simulink library "lib_all"  (Simulink 7.6)
settings: Solver=ode45  SolverMode=SingleTasking  StartTime=0.0  StopTime=10.0  RelTol=1e-3
values from neighbouring files (symbols this model uses):
  map_lib_03 = [6.8679 7.0159 7.4571 8.1849 9.1892 10.456 11.97 13.71 15.655 17.781 20.063 22.474 ... (803 elements, 73x11)]    [scr_simdata.mat]    (also in scr_simdata_all.mat)
  map_lib_06 = [2.5784 2.6727 2.9588 3.4453 4.1444 5.068 6.226 7.6242 9.2634 11.139 13.24 15.552 ... (803 elements, 73x11)]    [scr_simdata.mat]    (also in scr_simdata_all.mat)
  map_lib_09 = [0.0025784 0.0026727 0.0029588 0.0034453 0.0041444 0.005068 0.006226 0.0076242 0.0092634 0.011139 0.01324 0.015552 ... (803 elements, 73x11)]    [scr_simdata.mat]    (also in scr_simdata_all.mat)
  map_lib_10 = [2.74 2.75 2.76 2.77 2.78 2.79 2.8 2.81 2.82 2.83 2.84 2.85 ... (81 elements, 1x81)]    [scr_simdata.mat]    (also in scr_simdata_all.mat)
  map_lib_11 = [1.1983 1.2027 1.207 1.2113 1.2156 1.2198 1.2241 1.2283 1.2326 1.2368 1.241 1.2451 ... (81 elements, 1x81)]    [scr_simdata.mat]    (also in scr_simdata_all.mat)
  map_lib_12 = [2.74 2.75 2.76 2.77 2.78 2.79 2.8 2.81 2.82 2.83 2.84 2.85 ... (81 elements, 1x81)]    [scr_simdata.mat]    (also in scr_simdata_all.mat)
  map_lib_13 = [1.74 1.75 1.76 1.77 1.78 1.79 1.8 1.81 1.82 1.83 1.84 1.85 ... (81 elements, 1x81)]    [scr_simdata.mat]    (also in scr_simdata_all.mat)
  map_lib_16 = [0.00048828125 1.6159 1.8962 2.0821 2.2251 2.3426 2.4433 2.5318 2.611 2.683 2.749 2.8101 ... (1419 elements, 129x11)]    [scr_simdata.mat]    (also in scr_simdata_all.mat)
  map_mr2_01 = [0 800 1600 2400 3200 4000]    [scr_simdata.mat]    (also in scr_simdata_all.mat)
  map_mr2_02 = [1.32 1.32 1.33 1.34 1.36 1.38]    [scr_simdata.mat]    (also in scr_simdata_all.mat)
  map_mr2_03 = [0 800 1600 2400 3200 4000]    [scr_simdata.mat]    (also in scr_simdata_all.mat)
  map_mr2_04 = [1.32 1.32 1.33 1.34 1.36 1.38]    [scr_simdata.mat]    (also in scr_simdata_all.mat)
  map_mr2_05 = [0 800 1600 2400 3200 4000]    [scr_simdata.mat]    (also in scr_simdata_all.mat)
  map_mr2_06 = [1.32 1.32 1.33 1.34 1.36 1.38]    [scr_simdata.mat]    (also in scr_simdata_all.mat)
  Parameter1 = 6    [scr_simdata.mat]    (also in scr_simdata_all.mat)
  Parameter101 = 30    [scr_simdata.mat]    (also in scr_simdata_all.mat)
  Parameter102 = 12    [scr_simdata.mat]    (also in scr_simdata_all.mat)
  Parameter103 = -240    [scr_simdata.mat]    (also in scr_simdata_all.mat)
  Parameter108 = 48    [scr_simdata.mat]    (also in scr_simdata_all.mat)
  Parameter120 = 16    [scr_simdata.mat]    (also in scr_simdata_all.mat)
  Parameter121 = 87    [scr_simdata.mat]    (also in scr_simdata_all.mat)
  Parameter123 = 78    [scr_simdata.mat]    (also in scr_simdata_all.mat)
  Parameter124 = 60    [scr_simdata.mat]    (also in scr_simdata_all.mat)
  Parameter125 = -480    [scr_simdata.mat]    (also in scr_simdata_all.mat)
  Parameter130 = 96    [scr_simdata.mat]    (also in scr_simdata_all.mat)
  Parameter131 = 3    [scr_simdata.mat]    (also in scr_simdata_all.mat)
  Parameter144 = 3    [scr_simdata.mat]    (also in scr_simdata_all.mat)
  Parameter18 = 128    [scr_simdata.mat]    (also in scr_simdata_all.mat)
  Parameter19 = 16    [scr_simdata.mat]    (also in scr_simdata_all.mat)
  Parameter2 = 12    [scr_simdata.mat]    (also in scr_simdata_all.mat)
  Parameter20 = 7    [scr_simdata.mat]    (also in scr_simdata_all.mat)
  Parameter21 = 4    [scr_simdata.mat]    (also in scr_simdata_all.mat)
  Parameter3 = 18    [scr_simdata.mat]    (also in scr_simdata_all.mat)
  Parameter32 = 1    [scr_simdata.mat]    (also in scr_simdata_all.mat)
  Parameter34 = 24    [scr_simdata.mat]    (also in scr_simdata_all.mat)
  Parameter4 = 138    [scr_simdata.mat]    (also in scr_simdata_all.mat)
  Parameter42 = 48    [scr_simdata.mat]    (also in scr_simdata_all.mat)
  Parameter5 = 135    [scr_simdata.mat]    (also in scr_simdata_all.mat)
  Parameter55 = 0    [scr_simdata.mat]    (also in scr_simdata_all.mat)
  Parameter56 = 0    [scr_simdata.mat]    (also in scr_simdata_all.mat)
  Parameter57 = 1    [scr_simdata.mat]    (also in scr_simdata_all.mat)
  Parameter58 = 1    [scr_simdata.mat]    (also in scr_simdata_all.mat)
  Parameter59 = 2    [scr_simdata.mat]    (also in scr_simdata_all.mat)
  Parameter6 = 132    [scr_simdata.mat]    (also in scr_simdata_all.mat)
  Parameter60 = 2    [scr_simdata.mat]    (also in scr_simdata_all.mat)
  Parameter63 = 144    [scr_simdata.mat]    (also in scr_simdata_all.mat)
  Parameter7 = 126    [scr_simdata.mat]    (also in scr_simdata_all.mat)
  Parameter76 = 64    [scr_simdata.mat]    (also in scr_simdata_all.mat)
  Parameter77 = 135    [scr_simdata.mat]    (also in scr_simdata_all.mat)
  Parameter79 = 126    [scr_simdata.mat]    (also in scr_simdata_all.mat)
  Parameter80 = 108    [scr_simdata.mat]    (also in scr_simdata_all.mat)
  Parameter81 = 0    [scr_simdata.mat]    (also in scr_simdata_all.mat)
  Parameter86 = 0    [scr_simdata.mat]    (also in scr_simdata_all.mat)
  Parameter98 = 112    [scr_simdata.mat]    (also in scr_simdata_all.mat)
  Parameter99 = 39    [scr_simdata.mat]    (also in scr_simdata_all.mat)
undefined symbols (not defined in the file or in its folder): cylvolkp_hih_map2h, cylvolkp_map2h, pk1k_map2h
libraries: simulink x2
(part 5 of 36)

== system lib_all/SubSystem727/SubSystem737 ==
  Block1699 : Inport
  Block1700 : Inport  Port=2
  Block1701 : Inport  Port=3
  Block1702 : EnablePort
  Block1703 : DataStoreWrite  DataStoreName=DSM22
  Block1704 : DataStoreWrite  DataStoreName=DSM32
  Block1705 : DataStoreWrite  DataStoreName=DSM27
  wires:
  Block1699/1 -> Block1703/1
  Block1700/1 -> Block1704/1
  Block1701/1 -> Block1705/1

== system lib_all/SubSystem727/SubSystem738 ==
  Block1706 : Inport
  Block1707 : Inport  Port=2
  Block1708 : Inport  Port=3
  Block1709 : EnablePort
  Block1710 : DataStoreWrite  DataStoreName=DSM23
  Block1711 : DataStoreWrite  DataStoreName=DSM33
  Block1712 : DataStoreWrite  DataStoreName=DSM28
  wires:
  Block1706/1 -> Block1710/1
  Block1707/1 -> Block1711/1
  Block1708/1 -> Block1712/1

== system lib_all/SubSystem727/SubSystem739 ==
  Block1713 : Inport
  Block1714 : Inport  Port=2
  Block1715 : Inport  Port=3
  Block1716 : EnablePort
  Block1717 : DataStoreWrite  DataStoreName=DSM24
  Block1718 : DataStoreWrite  DataStoreName=DSM34
  Block1719 : DataStoreWrite  DataStoreName=DSM29
  wires:
  Block1715/1 -> Block1719/1
  Block1714/1 -> Block1718/1
  Block1713/1 -> Block1717/1

== system lib_all/SubSystem727/SubSystem740 ==
  note: Text113
  note: Text112
  Block1720 : Inport
  Block1721 : Inport  Port=2
  Block1722 : Inport  Port=3
  Block1723 : Constant  Value=0  OutDataTypeStr=double  SampleTime=-1
  SubSystem742 : Subsystem
  SubSystem743 : Subsystem
  SubSystem751 : Subsystem  TreatAsAtomicUnit=on
  SubSystem752 : Subsystem
  Block1778 : Outport
  wires:
  [TagName23] -> SubSystem751/3(Block1765)
  [TagName11] -> SubSystem751/2(Block1764)
  [TagName1] -> SubSystem751/1(Block1763)
  SubSystem742/1(Out1) -> SubSystem751/Enable
  Block1723/1 -> SubSystem742/2(In2)
  [TagName4] -> SubSystem742/1(In1)
  SubSystem752/1(Block1777) -> Block1778/1, [TagName23]
  Block1722/1 -> SubSystem752/1(Block1770)
  SubSystem743/1(Block1762) -> SubSystem752/2(Block1771), [TagName11]
  Block1720/1 -> SubSystem743/2(Block1733), [TagName4]
  Block1721/1 -> SubSystem743/1(Block1732), [TagName1]

== system lib_all/SubSystem727/SubSystem754 ==
  note: Text121
  note: Text120
  note: Text119
  Block1779 : Inport
  Block1780 : Inport  Port=2
  Block1781 : Inport  Port=3
  Block1782 : Constant  OutDataTypeStr=double  SampleTime=-1
  Block1783 : Gain  Gain=1/5  SaturateOnIntegerOverflow=on  OutDataTypeStr=Inherit: Same as input  ParamDataTypeStr=Inherit: Same as input
      settings: AttributesFormatString=Attribute365
  Block1784 : Product  OutDataTypeStr=double  SaturateOnIntegerOverflow=on  RndMeth=Zero  InputSameDT=on
  Block1785 : Sum  Inputs=-+  OutDataTypeStr=double  RndMeth=Zero  SaturateOnIntegerOverflow=on  InputSameDT=on
  Block1786 : Sum  OutDataTypeStr=double  RndMeth=Zero  SaturateOnIntegerOverflow=on  InputSameDT=on
  Block1787 : Sum  OutDataTypeStr=double  RndMeth=Zero  SaturateOnIntegerOverflow=on  InputSameDT=on
  SubSystem756 : Subsystem
  SubSystem762 : Subsystem
  Block1878 : Outport
  wires:
  Block1787/1 -> SubSystem762/3(Block1816), SubSystem756/2(Block1789)
  Block1779/1 -> Block1787/1
  Block1783/1 -> Block1786/2
  Block1784/1 -> Block1783/1
  Block1782/1 -> Block1787/2
  Block1786/1 -> Block1878/1
  SubSystem756/2(Block1813) -> Block1784/2
  Block1785/1 -> Block1784/1  "SigLabel48"
  SubSystem762/2(Block1877) -> Block1785/2
  SubSystem762/1(Block1876) -> Block1785/1, Block1786/1
  Block1781/1 -> SubSystem762/2(Block1815)
  SubSystem756/1(Block1812) -> SubSystem762/1(Block1814)
  Block1780/1 -> SubSystem756/1(Block1788)

== system lib_all/SubSystem795/SubSystem796 ==
  note: ***編集禁止***
  In1 : Inport
  In2 : Inport  Port=2
  RO1 : RelationalOperator  Operator=>  InputSameDT=on
  single1 : DataTypeConversion  OutDataTypeStr=single  SaturateOnIntegerOverflow=on  RndMeth=Zero
  single2 : DataTypeConversion  OutDataTypeStr=single  SaturateOnIntegerOverflow=on  RndMeth=Zero
  Out1 : Outport
  wires:
  RO1/1 -> Out1/1
  In2/1 -> single2/1
  In1/1 -> single1/1
  single1/1 -> RO1/1
  single2/1 -> RO1/2

== system lib_all/SubSystem795/SubSystem797 ==
  note: ***編集禁止***
  In1 : Inport
  In2 : Inport  Port=2
  RO1 : RelationalOperator  Operator=>  InputSameDT=on
  single1 : DataTypeConversion  OutDataTypeStr=single  SaturateOnIntegerOverflow=on  RndMeth=Zero
  single2 : DataTypeConversion  OutDataTypeStr=single  SaturateOnIntegerOverflow=on  RndMeth=Zero
  Out1 : Outport
  wires:
  single2/1 -> RO1/2
  single1/1 -> RO1/1
  In1/1 -> single1/1
  In2/1 -> single2/1
  RO1/1 -> Out1/1

== system lib_all/SubSystem795/SubSystem798 ==
  note: ***編集禁止***
  In1 : Inport
  In2 : Inport  Port=2
  LO1 : Logic
  RO1 : RelationalOperator  Operator=<  InputSameDT=on
  RO2 : RelationalOperator  Operator=~=  InputSameDT=on
  single1 : DataTypeConversion  OutDataTypeStr=single  SaturateOnIntegerOverflow=on  RndMeth=Zero
  single2 : DataTypeConversion  OutDataTypeStr=single  SaturateOnIntegerOverflow=on  RndMeth=Zero
  Out1 : Outport
  wires:
  single2/1 -> RO1/2, RO2/2
  single1/1 -> RO1/1, RO2/1
  In1/1 -> single1/1
  In2/1 -> single2/1
  RO1/1 -> LO1/1
  RO2/1 -> LO1/2
  LO1/1 -> Out1/1

== system lib_all/SubSystem795/SubSystem799 ==
  note: ***編集禁止***
  In1 : Inport
  In2 : Inport  Port=2
  LO1 : Logic
  RO1 : RelationalOperator  Operator=<  InputSameDT=on
  RO2 : RelationalOperator  Operator=~=  InputSameDT=on
  single1 : DataTypeConversion  OutDataTypeStr=single  SaturateOnIntegerOverflow=on  RndMeth=Zero
  single2 : DataTypeConversion  OutDataTypeStr=single  SaturateOnIntegerOverflow=on  RndMeth=Zero
  Out1 : Outport
  wires:
  single2/1 -> RO1/2, RO2/2
  single1/1 -> RO1/1, RO2/1
  In1/1 -> single1/1
  In2/1 -> single2/1
  RO1/1 -> LO1/1
  RO2/1 -> LO1/2
  LO1/1 -> Out1/1

== system lib_all/SubSystem800/SubSystem801 ==
  note: ***編集禁止***
  element : Inport
  Inside_DSR : DataStoreRead  DataStoreName=DSM182
  Selector : Selector  IndexOptions=Index vector (port)  Indices=[1 3]  OutputSizes=1
  Output : Outport
  wires:
  Inside_DSR/1 -> Selector/1
  element/1 -> Selector/2
  Selector/1 -> Output/1

== system lib_all/SubSystem800/SubSystem802 ==
  note: ***編集禁止***
  In1 : Inport
  In2 : Inport  Port=2
  LO1 : Logic
  RO1 : RelationalOperator  Operator=<  InputSameDT=on
  RO2 : RelationalOperator  Operator=~=  InputSameDT=on
  single1 : DataTypeConversion  OutDataTypeStr=single  SaturateOnIntegerOverflow=on  RndMeth=Zero
  single2 : DataTypeConversion  OutDataTypeStr=single  SaturateOnIntegerOverflow=on  RndMeth=Zero
  Out1 : Outport
  wires:
  In2/1 -> single2/1
  In1/1 -> single1/1
  single1/1 -> RO1/1, RO2/1
  single2/1 -> RO1/2, RO2/2
  RO1/1 -> LO1/1
  RO2/1 -> LO1/2
  LO1/1 -> Out1/1

== system lib_all/SubSystem800/SubSystem803 ==
  note: Text137
  note: Text136
  note: Text135
  note: Text134
  note: Text133
  Block1916 : Inport
  Block1917 : Inport  Port=2
  Block1918 : Constant  Value=Parameter81  OutDataTypeStr=double  SampleTime=-1
  Block1919 : Constant  Value=Parameter103  OutDataTypeStr=double  SampleTime=-1
  Block1920 : Constant  Value=Parameter125  OutDataTypeStr=double  SampleTime=-1
  Block1921 : Constant  Value=143  OutDataTypeStr=double  SampleTime=-1
  Block1922 : Constant  Value=-3  OutDataTypeStr=double  SampleTime=-1
  Block1923 : Constant  Value=359  OutDataTypeStr=double  SampleTime=-1
  Block1924 : Constant  Value=359  OutDataTypeStr=double  SampleTime=-1
  Block1925 : DataStoreRead  DataStoreName=DSM50
  Block1926 : Gain  Gain=5  SaturateOnIntegerOverflow=on  OutDataTypeStr=Inherit: Same as input  ParamDataTypeStr=Inherit: Same as input
      settings: AttributesFormatString=Attribute395
  Block1927 : Ground
  Block1928 : MultiPortSwitch  Inputs=4  zeroidx=off  RndMeth=Zero  SaturateOnIntegerOverflow=on  InputSameDT=on
  SubSystem804 : Subsystem  TreatAsAtomicUnit=on
      settings: AttributesFormatString=Attribute396  RTWFcnNameOpts=Use subsystem name
  SubSystem809 : Subsystem  TreatAsAtomicUnit=on
      settings: AttributesFormatString=Attribute403  RTWFcnNameOpts=Use subsystem name
  SubSystem814 : Subsystem  TreatAsAtomicUnit=on
      settings: AttributesFormatString=Attribute410  RTWFcnNameOpts=Use subsystem name
  Block2001 : Outport
  Block2002 : Outport  Port=2
  wires:
  Block1916/1 -> Block1928/1
  Block1918/1 -> Block1928/2
  Block1919/1 -> Block1928/3
  Block1920/1 -> Block1928/4
  Block1927/1 -> Block1928/5
  Block1928/1 -> SubSystem814/3(Block1979)  "SigLabel55"
  Block1921/1 -> SubSystem804/1(Block1929)
  Block1917/1 -> SubSystem804/2(Block1930), Block1926/1
  Block1922/1 -> SubSystem804/3(Block1931)
  Block1926/1 -> SubSystem809/2(Block1954)
  Block1923/1 -> SubSystem809/1(Block1953)
  Block1925/1 -> SubSystem809/3(Block1955)
  Block1924/1 -> SubSystem814/1(Block1977)
  SubSystem809/1(Block1976) -> SubSystem814/2(Block1978)
  SubSystem804/1(Block1952) -> Block2001/1
  SubSystem814/1(Block2000) -> Block2002/1

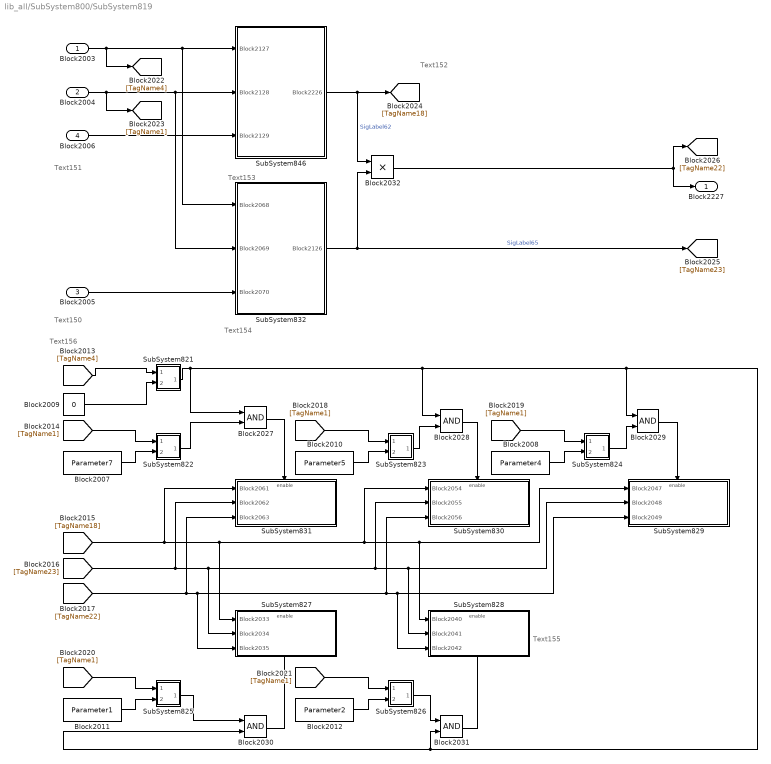
== system lib_all/SubSystem800/SubSystem819 ==
  note: Text156
  note: Text155
  note: Text154
  note: Text153
  note: Text152
  note: Text151
  note: Text150
  Block2003 : Inport
  Block2004 : Inport  Port=2
  Block2005 : Inport  Port=3
  Block2006 : Inport  Port=4
  Block2007 : Constant  Value=Parameter7  OutDataTypeStr=double  SampleTime=-1
  Block2008 : Constant  Value=Parameter4  OutDataTypeStr=double  SampleTime=-1
  Block2009 : Constant  Value=0  OutDataTypeStr=double  SampleTime=-1
  Block2010 : Constant  Value=Parameter5  OutDataTypeStr=double  SampleTime=-1
  Block2011 : Constant  Value=Parameter1  OutDataTypeStr=double  SampleTime=-1
  Block2012 : Constant  Value=Parameter2  OutDataTypeStr=double  SampleTime=-1
  Block{2027..2031} (5 alike) : Logic
  Block2032 : Product  OutDataTypeStr=double  SaturateOnIntegerOverflow=on  RndMeth=Zero  InputSameDT=on
  SubSystem821 : Subsystem
  SubSystem822 : Subsystem
  SubSystem823 : Subsystem
  SubSystem824 : Subsystem
  SubSystem825 : Subsystem
  SubSystem826 : Subsystem
  SubSystem827 : Subsystem
      settings: PropExecContextOutsideSubsystem=on
  SubSystem828 : Subsystem
      settings: PropExecContextOutsideSubsystem=on
  SubSystem829 : Subsystem
      settings: PropExecContextOutsideSubsystem=on
  SubSystem830 : Subsystem
      settings: PropExecContextOutsideSubsystem=on
  SubSystem831 : Subsystem
      settings: PropExecContextOutsideSubsystem=on
  SubSystem832 : Subsystem  Priority=2  TreatAsAtomicUnit=on
      settings: AttributesFormatString=Attribute424  RTWFcnNameOpts=Use subsystem name
  SubSystem846 : Subsystem  Priority=1  TreatAsAtomicUnit=on
      settings: AttributesFormatString=Attribute438  RTWFcnNameOpts=Use subsystem name
  Block2227 : Outport
  wires:
  SubSystem826/1(Out1) -> Block2031/1
  SubSystem825/1(Out1) -> Block2030/1
  Block2031/1 -> SubSystem828/Enable
  Block2030/1 -> SubSystem827/Enable
  Block2012/1 -> SubSystem826/2(In2)
  [TagName1] -> SubSystem826/1(In1)
  Block2011/1 -> SubSystem825/2(In2)
  [TagName1] -> SubSystem825/1(In1)
  [TagName22] -> SubSystem831/3(Block2063), SubSystem827/3(Block2035), SubSystem829/3(Block2049), SubSystem828/3(Block2042), SubSystem830/3(Block2056)
  [TagName23] -> SubSystem831/2(Block2062), SubSystem827/2(Block2034), SubSystem829/2(Block2048), SubSystem828/2(Block2041), SubSystem830/2(Block2055)
  [TagName18] -> SubSystem831/1(Block2061), SubSystem827/1(Block2033), SubSystem829/1(Block2047), SubSystem828/1(Block2040), SubSystem830/1(Block2054)
  Block2029/1 -> SubSystem829/Enable
  Block2028/1 -> SubSystem830/Enable
  SubSystem824/1(Out1) -> Block2029/2
  Block2008/1 -> SubSystem824/2(In2)
  [TagName1] -> SubSystem824/1(In1)
  Block2027/1 -> SubSystem831/Enable
  SubSystem823/1(Out1) -> Block2028/2
  Block2010/1 -> SubSystem823/2(In2)
  [TagName1] -> SubSystem823/1(In1)
  SubSystem822/1(Out1) -> Block2027/2
  SubSystem821/1(Out1) -> Block2027/1, Block2028/1, Block2029/1, Block2031/2, Block2030/2
  Block2007/1 -> SubSystem822/2(In2)
  [TagName1] -> SubSystem822/1(In1)
  Block2009/1 -> SubSystem821/2(In2)
  [TagName4] -> SubSystem821/1(In1)
  Block2032/1 -> Block2227/1, [TagName22]
  SubSystem832/1(Block2126) -> Block2032/2, [TagName23]  "SigLabel65"
  SubSystem846/1(Block2226) -> Block2032/1, [TagName18]  "SigLabel62"
  Block2004/1 -> SubSystem832/2(Block2069), SubSystem846/2(Block2128), [TagName1]
  Block2003/1 -> SubSystem832/1(Block2068), SubSystem846/1(Block2127), [TagName4]
  Block2006/1 -> SubSystem846/3(Block2129)
  Block2005/1 -> SubSystem832/3(Block2070)

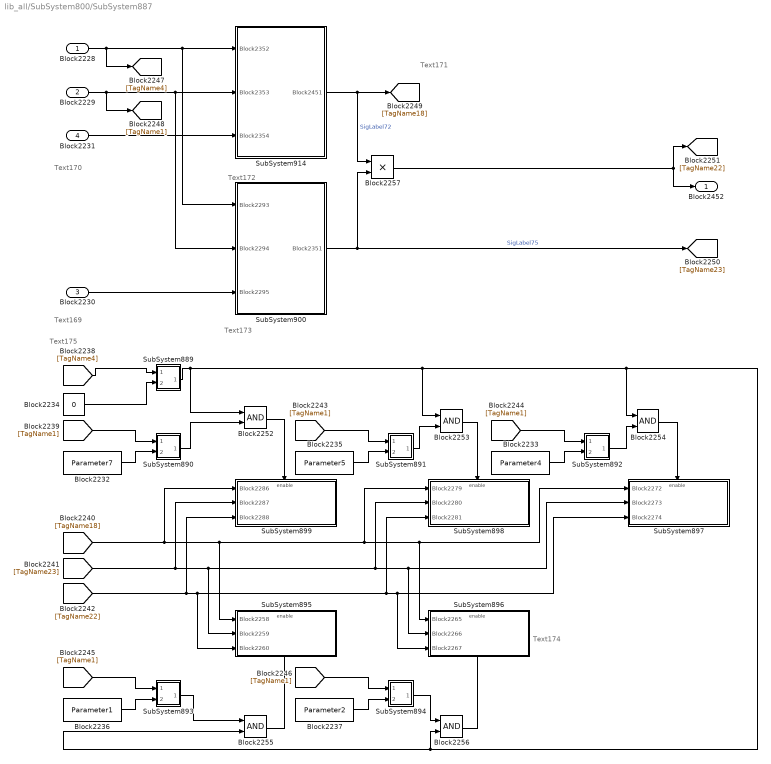
== system lib_all/SubSystem800/SubSystem887 ==
  note: Text175
  note: Text174
  note: Text173
  note: Text172
  note: Text171
  note: Text170
  note: Text169
  Block2228 : Inport
  Block2229 : Inport  Port=2
  Block2230 : Inport  Port=3
  Block2231 : Inport  Port=4
  Block2232 : Constant  Value=Parameter7  OutDataTypeStr=double  SampleTime=-1
  Block2233 : Constant  Value=Parameter4  OutDataTypeStr=double  SampleTime=-1
  Block2234 : Constant  Value=0  OutDataTypeStr=double  SampleTime=-1
  Block2235 : Constant  Value=Parameter5  OutDataTypeStr=double  SampleTime=-1
  Block2236 : Constant  Value=Parameter1  OutDataTypeStr=double  SampleTime=-1
  Block2237 : Constant  Value=Parameter2  OutDataTypeStr=double  SampleTime=-1
  Block{2252..2256} (5 alike) : Logic
  Block2257 : Product  OutDataTypeStr=double  SaturateOnIntegerOverflow=on  RndMeth=Zero  InputSameDT=on
  SubSystem889 : Subsystem
  SubSystem890 : Subsystem
  SubSystem891 : Subsystem
  SubSystem892 : Subsystem
  SubSystem893 : Subsystem
  SubSystem894 : Subsystem
  SubSystem895 : Subsystem
      settings: PropExecContextOutsideSubsystem=on
  SubSystem896 : Subsystem
      settings: PropExecContextOutsideSubsystem=on
  SubSystem897 : Subsystem
      settings: PropExecContextOutsideSubsystem=on
  SubSystem898 : Subsystem
      settings: PropExecContextOutsideSubsystem=on
  SubSystem899 : Subsystem
      settings: PropExecContextOutsideSubsystem=on
  SubSystem900 : Subsystem  Priority=2  TreatAsAtomicUnit=on
      settings: AttributesFormatString=Attribute461  RTWFcnNameOpts=Use subsystem name
  SubSystem914 : Subsystem  Priority=1  TreatAsAtomicUnit=on
      settings: AttributesFormatString=Attribute475  RTWFcnNameOpts=Use subsystem name
  Block2452 : Outport
  wires:
  SubSystem894/1(Out1) -> Block2256/1
  SubSystem893/1(Out1) -> Block2255/1
  Block2256/1 -> SubSystem896/Enable
  Block2255/1 -> SubSystem895/Enable
  Block2237/1 -> SubSystem894/2(In2)
  [TagName1] -> SubSystem894/1(In1)
  Block2236/1 -> SubSystem893/2(In2)
  [TagName1] -> SubSystem893/1(In1)
  [TagName22] -> SubSystem899/3(Block2288), SubSystem895/3(Block2260), SubSystem897/3(Block2274), SubSystem896/3(Block2267), SubSystem898/3(Block2281)
  [TagName23] -> SubSystem899/2(Block2287), SubSystem895/2(Block2259), SubSystem897/2(Block2273), SubSystem896/2(Block2266), SubSystem898/2(Block2280)
  [TagName18] -> SubSystem899/1(Block2286), SubSystem895/1(Block2258), SubSystem897/1(Block2272), SubSystem896/1(Block2265), SubSystem898/1(Block2279)
  Block2254/1 -> SubSystem897/Enable
  Block2253/1 -> SubSystem898/Enable
  SubSystem892/1(Out1) -> Block2254/2
  Block2233/1 -> SubSystem892/2(In2)
  [TagName1] -> SubSystem892/1(In1)
  Block2252/1 -> SubSystem899/Enable
  SubSystem891/1(Out1) -> Block2253/2
  Block2235/1 -> SubSystem891/2(In2)
  [TagName1] -> SubSystem891/1(In1)
  SubSystem890/1(Out1) -> Block2252/2
  SubSystem889/1(Out1) -> Block2252/1, Block2253/1, Block2254/1, Block2256/2, Block2255/2
  Block2232/1 -> SubSystem890/2(In2)
  [TagName1] -> SubSystem890/1(In1)
  Block2234/1 -> SubSystem889/2(In2)
  [TagName4] -> SubSystem889/1(In1)
  Block2257/1 -> Block2452/1, [TagName22]
  SubSystem900/1(Block2351) -> Block2257/2, [TagName23]  "SigLabel75"
  SubSystem914/1(Block2451) -> Block2257/1, [TagName18]  "SigLabel72"
  Block2229/1 -> SubSystem900/2(Block2294), SubSystem914/2(Block2353), [TagName1]
  Block2228/1 -> SubSystem900/1(Block2293), SubSystem914/1(Block2352), [TagName4]
  Block2231/1 -> SubSystem914/3(Block2354)
  Block2230/1 -> SubSystem900/3(Block2295)

== system lib_all/SubSystem800/SubSystem955 ==
  Block2453 : Inport
  Block2454 : Inport  Port=2
  Block2455 : Inport  Port=3
  Block2456 : Inport  Port=4
  Block2457 : EnablePort
  SubSystem956 : Subsystem  TreatAsAtomicUnit=on  mask(***ATC.DSWVEC***){bd_version=1.01.A, r0=DSM182, r1=-1, r2=, r3=, r4=, r5=, r6=, r7=, r8=, r9=, c0=, c1=, c2=, c3=, c4=, c5=, c6=, c7=, c8=, c9=}
      mask prompts: bd_version "Version"
      mask help: これはAutoCからのブロックです．編集禁止．
      settings: OpenFcn=warndlg('変更禁止!');
      mask Help: web(ui_blockhelpmng(gcb));
      mask Display: disp('ATC.DSRVEC')
  SubSystem957 : Subsystem  TreatAsAtomicUnit=on  mask(***ATC.DSWVEC***){bd_version=1.01.A, r0=DSM184, r1=-1, r2=, r3=, r4=, r5=, r6=, r7=, r8=, r9=, c0=, c1=, c2=, c3=, c4=, c5=, c6=, c7=, c8=, c9=}
      mask prompts: bd_version "Version"
      mask help: これはAutoCからのブロックです．編集禁止．
      settings: OpenFcn=warndlg('変更禁止!');
      mask Help: web(ui_blockhelpmng(gcb));
      mask Display: disp('ATC.DSRVEC')
  SubSystem958 : Subsystem  TreatAsAtomicUnit=on  mask(***ATC.DSWVEC***){bd_version=1.01.A, r0=DSM183, r1=-1, r2=, r3=, r4=, r5=, r6=, r7=, r8=, r9=, c0=, c1=, c2=, c3=, c4=, c5=, c6=, c7=, c8=, c9=}
      mask prompts: bd_version "Version"
      mask help: これはAutoCからのブロックです．編集禁止．
      settings: OpenFcn=warndlg('変更禁止!');
      mask Help: web(ui_blockhelpmng(gcb));
      mask Display: disp('ATC.DSRVEC')
  wires:
  Block2456/1 -> SubSystem958/1(input)
  Block2455/1 -> SubSystem957/1(input)
  Block2454/1 -> SubSystem956/1(input)
  Block2453/1 -> SubSystem957/2(element), SubSystem958/2(element), SubSystem956/2(element)

== system lib_all/SubSystem959/SubSystem961 ==
  note: ***編集禁止***
  N : Inport
  For_loop : Stateflow chart  TreatAsAtomicUnit=on
  action : Outport
  i : Outport  Port=2
  wires:
  For_loop/2(function_call) -> action/1
  N/1 -> For_loop/1(N_Loop)
  For_loop/1(idx) -> i/1

== system lib_all/SubSystem959/SubSystem963 ==
  note: Text186
  Block2469 : Inport
  Block2470 : Inport  Port=2
  Block2471 : Inport  Port=3
  Block2472 : TriggerPort  TriggerType=function-call
  Block2473 : Constant  Value=2  OutDataTypeStr=double  SampleTime=-1
  Block2474 : Constant  OutDataTypeStr=double  SampleTime=-1
  Block2475 : Constant  Value=144  OutDataTypeStr=double  SampleTime=-1
  Block2476 : Constant  Value=0  OutDataTypeStr=double  SampleTime=-1
  Block2477 : Constant  Value=0  OutDataTypeStr=double  SampleTime=-1
  Block2478 : Constant  OutDataTypeStr=double  SampleTime=-1
  Block2479 : Constant  OutDataTypeStr=double  SampleTime=-1
  Block2480 : Sum  Inputs=+-  OutDataTypeStr=double  RndMeth=Zero  SaturateOnIntegerOverflow=on  InputSameDT=on
  Block{2481..2485} (5 alike) : Sum  OutDataTypeStr=double  RndMeth=Zero  SaturateOnIntegerOverflow=on  InputSameDT=on
  Block2486 : Switch  Threshold=0.5  RndMeth=Zero  SaturateOnIntegerOverflow=on  InputSameDT=on
  Block2487 : Switch  Threshold=0.5  RndMeth=Zero  SaturateOnIntegerOverflow=on  InputSameDT=on
  Block2488 : UnitDelay  SampleTime=-1
  Block2489 : UnitDelay  SampleTime=-1
  SubSystem1018 : Subsystem
      settings: RTWSystemCode=Inline
  SubSystem1021 : Subsystem  TreatAsAtomicUnit=on  mask(***ATC.DSRVEC***){bd_version=1.01.A, r0=DSM49, r1=-1, r2=, r3=, r4=, r5=, r6=, r7=, r8=, r9=, c0=, c1=, c2=, c3=, c4=, c5=, c6=, c7=, c8=, c9=}
      mask prompts: bd_version "Version"
      mask help: これはAutoCからのブロックです．編集禁止．
      settings: OpenFcn=warndlg('変更禁止!');
      mask Help: web(ui_blockhelpmng(gcb));
      mask Display: disp('ATC.DSRVEC')
  SubSystem1022 : Subsystem  TreatAsAtomicUnit=on  mask(***ATC.DSRVEC***){bd_version=1.01.A, r0=DSM36, r1=-1, r2=, r3=, r4=, r5=, r6=, r7=, r8=, r9=, c0=, c1=, c2=, c3=, c4=, c5=, c6=, c7=, c8=, c9=}
      mask prompts: bd_version "Version"
      mask help: これはAutoCからのブロックです．編集禁止．
      settings: OpenFcn=warndlg('変更禁止!');
      mask Help: web(ui_blockhelpmng(gcb));
      mask Display: disp('ATC.DSRVEC')
  SubSystem964 : Subsystem
  SubSystem965 : Subsystem  TreatAsAtomicUnit=on
      settings: AttributesFormatString=Attribute504  RTWFcnNameOpts=Use subsystem name
  Block2620 : Outport
  Block2621 : Outport  Port=2
  wires:
  Block2486/1 -> Block2620/1, Block2489/1
  Block2473/1 -> Block2480/2
  Block2480/1 -> Block2481/1
  Block2481/1 -> SubSystem1018/2(Block2613)
  Block2475/1 -> SubSystem1018/1(Block2612)
  Block2476/1 -> SubSystem1018/3(Block2614)
  Block2471/1 -> SubSystem965/1(Block2490), Block2485/1
  Block2477/1 -> SubSystem965/4(Block2493)
  SubSystem1022/1(Output) -> SubSystem965/3(Block2492)
  Block2469/1 -> Block2480/1, SubSystem964/2(In2)
  Block2470/1 -> Block2481/2
  SubSystem965/1(Block2611) -> Block2487/1, Block2482/1
  Block2488/1 -> Block2482/2
  SubSystem1021/1(Output) -> Block2486/1, Block2483/1
  Block2489/1 -> Block2483/2
  SubSystem1018/1(Block2619) -> SubSystem965/2(Block2491), Block2484/1
  Block2485/1 -> SubSystem1022/1(element)
  Block2484/1 -> SubSystem1021/1(element)
  Block2474/1 -> SubSystem964/1(In1)
  SubSystem964/1(Out1) -> Block2486/2, Block2487/2
  Block2483/1 -> Block2486/3
  Block2482/1 -> Block2487/3
  Block2487/1 -> Block2621/1, Block2488/1
  Block2478/1 -> Block2484/2
  Block2479/1 -> Block2485/2

== system lib_all/SubSystem1023/SubSystem1025/SubSystem1027 ==
  note: ***編集禁止***
  In1 : Inport
  In2 : Inport  Port=2
  RO1 : RelationalOperator  Operator===  InputSameDT=on
  single1 : DataTypeConversion  OutDataTypeStr=single  SaturateOnIntegerOverflow=on  RndMeth=Zero
  single2 : DataTypeConversion  OutDataTypeStr=single  SaturateOnIntegerOverflow=on  RndMeth=Zero
  Out1 : Outport
  wires:
  single2/1 -> RO1/2
  single1/1 -> RO1/1
  In1/1 -> single1/1
  In2/1 -> single2/1
  RO1/1 -> Out1/1

== system lib_all/SubSystem1023/SubSystem1025/SubSystem1028 ==
  note: ***編集禁止***
  In1 : Inport
  In2 : Inport  Port=2
  RO1 : RelationalOperator  Operator===  InputSameDT=on
  single1 : DataTypeConversion  OutDataTypeStr=single  SaturateOnIntegerOverflow=on  RndMeth=Zero
  single2 : DataTypeConversion  OutDataTypeStr=single  SaturateOnIntegerOverflow=on  RndMeth=Zero
  Out1 : Outport
  wires:
  single2/1 -> RO1/2
  single1/1 -> RO1/1
  In1/1 -> single1/1
  In2/1 -> single2/1
  RO1/1 -> Out1/1

== system lib_all/SubSystem1023/SubSystem1025/SubSystem1029 ==
  note: ***編集禁止***
  In1 : Inport
  In2 : Inport  Port=2
  RO1 : RelationalOperator  Operator===  InputSameDT=on
  single1 : DataTypeConversion  OutDataTypeStr=single  SaturateOnIntegerOverflow=on  RndMeth=Zero
  single2 : DataTypeConversion  OutDataTypeStr=single  SaturateOnIntegerOverflow=on  RndMeth=Zero
  Out1 : Outport
  wires:
  single2/1 -> RO1/2
  single1/1 -> RO1/1
  In1/1 -> single1/1
  In2/1 -> single2/1
  RO1/1 -> Out1/1

== system lib_all/SubSystem1023/SubSystem1025/SubSystem1030 ==
  note: ***編集禁止***
  In1 : Inport
  In2 : Inport  Port=2
  RO1 : RelationalOperator  Operator===  InputSameDT=on
  single1 : DataTypeConversion  OutDataTypeStr=single  SaturateOnIntegerOverflow=on  RndMeth=Zero
  single2 : DataTypeConversion  OutDataTypeStr=single  SaturateOnIntegerOverflow=on  RndMeth=Zero
  Out1 : Outport
  wires:
  single2/1 -> RO1/2
  single1/1 -> RO1/1
  In1/1 -> single1/1
  In2/1 -> single2/1
  RO1/1 -> Out1/1

== system lib_all/SubSystem1023/SubSystem1025/SubSystem1031 ==
  note: ***編集禁止***
  In1 : Inport
  In2 : Inport  Port=2
  RO1 : RelationalOperator  Operator===  InputSameDT=on
  single1 : DataTypeConversion  OutDataTypeStr=single  SaturateOnIntegerOverflow=on  RndMeth=Zero
  single2 : DataTypeConversion  OutDataTypeStr=single  SaturateOnIntegerOverflow=on  RndMeth=Zero
  Out1 : Outport
  wires:
  single2/1 -> RO1/2
  single1/1 -> RO1/1
  In1/1 -> single1/1
  In2/1 -> single2/1
  RO1/1 -> Out1/1

== system lib_all/SubSystem1023/SubSystem1025/SubSystem1032 ==
  note: ***編集禁止***
  In1 : Inport
  In2 : Inport  Port=2
  RO1 : RelationalOperator  Operator===  InputSameDT=on
  single1 : DataTypeConversion  OutDataTypeStr=single  SaturateOnIntegerOverflow=on  RndMeth=Zero
  single2 : DataTypeConversion  OutDataTypeStr=single  SaturateOnIntegerOverflow=on  RndMeth=Zero
  Out1 : Outport
  wires:
  single2/1 -> RO1/2
  single1/1 -> RO1/1
  In1/1 -> single1/1
  In2/1 -> single2/1
  RO1/1 -> Out1/1

== system lib_all/SubSystem1023/SubSystem1025/SubSystem1033 ==
  Block2660 : Inport
  Block2661 : Inport  Port=2
  Block2662 : Inport  Port=3
  Block2663 : EnablePort
  Block2664 : DataStoreWrite  DataStoreName=DSM20
  Block2665 : DataStoreWrite  DataStoreName=DSM30
  Block2666 : DataStoreWrite  DataStoreName=DSM25
  wires:
  Block2660/1 -> Block2664/1
  Block2661/1 -> Block2665/1
  Block2662/1 -> Block2666/1

== system lib_all/SubSystem1023/SubSystem1025/SubSystem1034 ==
  Block2667 : Inport
  Block2668 : Inport  Port=2
  Block2669 : Inport  Port=3
  Block2670 : EnablePort
  Block2671 : DataStoreWrite  DataStoreName=DSM21
  Block2672 : DataStoreWrite  DataStoreName=DSM31
  Block2673 : DataStoreWrite  DataStoreName=DSM26
  wires:
  Block2667/1 -> Block2671/1
  Block2668/1 -> Block2672/1
  Block2669/1 -> Block2673/1

== system lib_all/SubSystem1023/SubSystem1025/SubSystem1035 ==
  Block2674 : Inport
  Block2675 : Inport  Port=2
  Block2676 : Inport  Port=3
  Block2677 : EnablePort
  Block2678 : DataStoreWrite  DataStoreName=DSM22
  Block2679 : DataStoreWrite  DataStoreName=DSM32
  Block2680 : DataStoreWrite  DataStoreName=DSM27
  wires:
  Block2674/1 -> Block2678/1
  Block2675/1 -> Block2679/1
  Block2676/1 -> Block2680/1

== system lib_all/SubSystem1023/SubSystem1025/SubSystem1036 ==
  Block2681 : Inport
  Block2682 : Inport  Port=2
  Block2683 : Inport  Port=3
  Block2684 : EnablePort
  Block2685 : DataStoreWrite  DataStoreName=DSM23
  Block2686 : DataStoreWrite  DataStoreName=DSM33
  Block2687 : DataStoreWrite  DataStoreName=DSM28
  wires:
  Block2681/1 -> Block2685/1
  Block2682/1 -> Block2686/1
  Block2683/1 -> Block2687/1

== system lib_all/SubSystem1023/SubSystem1025/SubSystem1037 ==
  Block2688 : Inport
  Block2689 : Inport  Port=2
  Block2690 : Inport  Port=3
  Block2691 : EnablePort
  Block2692 : DataStoreWrite  DataStoreName=DSM24
  Block2693 : DataStoreWrite  DataStoreName=DSM34
  Block2694 : DataStoreWrite  DataStoreName=DSM29
  wires:
  Block2690/1 -> Block2694/1
  Block2689/1 -> Block2693/1
  Block2688/1 -> Block2692/1

== system lib_all/SubSystem1023/SubSystem1025/SubSystem1038 ==
  note: Text192
  note: Text191
  Block2695 : Inport
  Block2696 : Inport  Port=2
  Block2697 : Inport  Port=3
  Block2698 : Constant  Value=0  OutDataTypeStr=double  SampleTime=-1
  SubSystem1040 : Subsystem
  SubSystem1041 : Subsystem
  SubSystem1049 : Subsystem  TreatAsAtomicUnit=on
  SubSystem1050 : Subsystem
  Block2753 : Outport
  wires:
  Block2696/1 -> [TagName1], SubSystem1041/1(Block2707)
  Block2695/1 -> [TagName4], SubSystem1041/2(Block2708)
  SubSystem1041/1(Block2737) -> [TagName11], SubSystem1050/2(Block2746)
  Block2697/1 -> SubSystem1050/1(Block2745)
  SubSystem1050/1(Block2752) -> [TagName23], Block2753/1
  [TagName4] -> SubSystem1040/1(In1)
  Block2698/1 -> SubSystem1040/2(In2)
  SubSystem1040/1(Out1) -> SubSystem1049/Enable
  [TagName1] -> SubSystem1049/1(Block2738)
  [TagName11] -> SubSystem1049/2(Block2739)
  [TagName23] -> SubSystem1049/3(Block2740)

== system lib_all/SubSystem1023/SubSystem1025/SubSystem1052 ==
  note: Text200
  note: Text199
  note: Text198
  Block2754 : Inport
  Block2755 : Inport  Port=2
  Block2756 : Inport  Port=3
  Block2757 : Constant  OutDataTypeStr=double  SampleTime=-1
  Block2758 : Gain  Gain=1/5  SaturateOnIntegerOverflow=on  OutDataTypeStr=Inherit: Same as input  ParamDataTypeStr=Inherit: Same as input
      settings: AttributesFormatString=Attribute549
  Block2759 : Product  OutDataTypeStr=double  SaturateOnIntegerOverflow=on  RndMeth=Zero  InputSameDT=on
  Block2760 : Sum  Inputs=-+  OutDataTypeStr=double  RndMeth=Zero  SaturateOnIntegerOverflow=on  InputSameDT=on
  Block2761 : Sum  OutDataTypeStr=double  RndMeth=Zero  SaturateOnIntegerOverflow=on  InputSameDT=on
  Block2762 : Sum  OutDataTypeStr=double  RndMeth=Zero  SaturateOnIntegerOverflow=on  InputSameDT=on
  SubSystem1054 : Subsystem
  SubSystem1060 : Subsystem
  Block2853 : Outport
  wires:
  Block2755/1 -> SubSystem1054/1(Block2763)
  SubSystem1054/1(Block2787) -> SubSystem1060/1(Block2789)
  Block2756/1 -> SubSystem1060/2(Block2790)
  SubSystem1060/1(Block2851) -> Block2761/1, Block2760/1
  SubSystem1060/2(Block2852) -> Block2760/2
  Block2760/1 -> Block2759/1  "SigLabel89"
  SubSystem1054/2(Block2788) -> Block2759/2
  Block2761/1 -> Block2853/1
  Block2757/1 -> Block2762/2
  Block2759/1 -> Block2758/1
  Block2758/1 -> Block2761/2
  Block2754/1 -> Block2762/1
  Block2762/1 -> SubSystem1054/2(Block2764), SubSystem1060/3(Block2791)

== system lib_all/SubSystem1023/SubSystem1093/SubSystem1095 ==
  note: ***編集禁止***
  In1 : Inport
  In2 : Inport  Port=2
  RO1 : RelationalOperator  Operator===  InputSameDT=on
  single1 : DataTypeConversion  OutDataTypeStr=single  SaturateOnIntegerOverflow=on  RndMeth=Zero
  single2 : DataTypeConversion  OutDataTypeStr=single  SaturateOnIntegerOverflow=on  RndMeth=Zero
  Out1 : Outport
  wires:
  single2/1 -> RO1/2
  single1/1 -> RO1/1
  In1/1 -> single1/1
  In2/1 -> single2/1
  RO1/1 -> Out1/1

== system lib_all/SubSystem1023/SubSystem1093/SubSystem1096 ==
  note: ***編集禁止***
  In1 : Inport
  In2 : Inport  Port=2
  RO1 : RelationalOperator  Operator===  InputSameDT=on
  single1 : DataTypeConversion  OutDataTypeStr=single  SaturateOnIntegerOverflow=on  RndMeth=Zero
  single2 : DataTypeConversion  OutDataTypeStr=single  SaturateOnIntegerOverflow=on  RndMeth=Zero
  Out1 : Outport
  wires:
  single2/1 -> RO1/2
  single1/1 -> RO1/1
  In1/1 -> single1/1
  In2/1 -> single2/1
  RO1/1 -> Out1/1

== system lib_all/SubSystem1023/SubSystem1093/SubSystem1097 ==
  note: ***編集禁止***
  In1 : Inport
  In2 : Inport  Port=2
  RO1 : RelationalOperator  Operator===  InputSameDT=on
  single1 : DataTypeConversion  OutDataTypeStr=single  SaturateOnIntegerOverflow=on  RndMeth=Zero
  single2 : DataTypeConversion  OutDataTypeStr=single  SaturateOnIntegerOverflow=on  RndMeth=Zero
  Out1 : Outport
  wires:
  single2/1 -> RO1/2
  single1/1 -> RO1/1
  In1/1 -> single1/1
  In2/1 -> single2/1
  RO1/1 -> Out1/1

== system lib_all/SubSystem1023/SubSystem1093/SubSystem1098 ==
  note: ***編集禁止***
  In1 : Inport
  In2 : Inport  Port=2
  RO1 : RelationalOperator  Operator===  InputSameDT=on
  single1 : DataTypeConversion  OutDataTypeStr=single  SaturateOnIntegerOverflow=on  RndMeth=Zero
  single2 : DataTypeConversion  OutDataTypeStr=single  SaturateOnIntegerOverflow=on  RndMeth=Zero
  Out1 : Outport
  wires:
  single2/1 -> RO1/2
  single1/1 -> RO1/1
  In1/1 -> single1/1
  In2/1 -> single2/1
  RO1/1 -> Out1/1

== system lib_all/SubSystem1023/SubSystem1093/SubSystem1099 ==
  note: ***編集禁止***
  In1 : Inport
  In2 : Inport  Port=2
  RO1 : RelationalOperator  Operator===  InputSameDT=on
  single1 : DataTypeConversion  OutDataTypeStr=single  SaturateOnIntegerOverflow=on  RndMeth=Zero
  single2 : DataTypeConversion  OutDataTypeStr=single  SaturateOnIntegerOverflow=on  RndMeth=Zero
  Out1 : Outport
  wires:
  single2/1 -> RO1/2
  single1/1 -> RO1/1
  In1/1 -> single1/1
  In2/1 -> single2/1
  RO1/1 -> Out1/1

== system lib_all/SubSystem1023/SubSystem1093/SubSystem1100 ==
  note: ***編集禁止***
  In1 : Inport
  In2 : Inport  Port=2
  RO1 : RelationalOperator  Operator===  InputSameDT=on
  single1 : DataTypeConversion  OutDataTypeStr=single  SaturateOnIntegerOverflow=on  RndMeth=Zero
  single2 : DataTypeConversion  OutDataTypeStr=single  SaturateOnIntegerOverflow=on  RndMeth=Zero
  Out1 : Outport
  wires:
  single2/1 -> RO1/2
  single1/1 -> RO1/1
  In1/1 -> single1/1
  In2/1 -> single2/1
  RO1/1 -> Out1/1

== system lib_all/SubSystem1023/SubSystem1093/SubSystem1101 ==
  Block2885 : Inport
  Block2886 : Inport  Port=2
  Block2887 : Inport  Port=3
  Block2888 : EnablePort
  Block2889 : DataStoreWrite  DataStoreName=DSM20
  Block2890 : DataStoreWrite  DataStoreName=DSM30
  Block2891 : DataStoreWrite  DataStoreName=DSM25
  wires:
  Block2885/1 -> Block2889/1
  Block2886/1 -> Block2890/1
  Block2887/1 -> Block2891/1

== system lib_all/SubSystem1023/SubSystem1093/SubSystem1102 ==
  Block2892 : Inport
  Block2893 : Inport  Port=2
  Block2894 : Inport  Port=3
  Block2895 : EnablePort
  Block2896 : DataStoreWrite  DataStoreName=DSM21
  Block2897 : DataStoreWrite  DataStoreName=DSM31
  Block2898 : DataStoreWrite  DataStoreName=DSM26
  wires:
  Block2892/1 -> Block2896/1
  Block2893/1 -> Block2897/1
  Block2894/1 -> Block2898/1

== system lib_all/SubSystem1023/SubSystem1093/SubSystem1103 ==
  Block2899 : Inport
  Block2900 : Inport  Port=2
  Block2901 : Inport  Port=3
  Block2902 : EnablePort
  Block2903 : DataStoreWrite  DataStoreName=DSM22
  Block2904 : DataStoreWrite  DataStoreName=DSM32
  Block2905 : DataStoreWrite  DataStoreName=DSM27
  wires:
  Block2899/1 -> Block2903/1
  Block2900/1 -> Block2904/1
  Block2901/1 -> Block2905/1

== system lib_all/SubSystem1023/SubSystem1093/SubSystem1104 ==
  Block2906 : Inport
  Block2907 : Inport  Port=2
  Block2908 : Inport  Port=3
  Block2909 : EnablePort
  Block2910 : DataStoreWrite  DataStoreName=DSM23
  Block2911 : DataStoreWrite  DataStoreName=DSM33
  Block2912 : DataStoreWrite  DataStoreName=DSM28
  wires:
  Block2906/1 -> Block2910/1
  Block2907/1 -> Block2911/1
  Block2908/1 -> Block2912/1

== system lib_all/SubSystem1023/SubSystem1093/SubSystem1105 ==
  Block2913 : Inport
  Block2914 : Inport  Port=2
  Block2915 : Inport  Port=3
  Block2916 : EnablePort
  Block2917 : DataStoreWrite  DataStoreName=DSM24
  Block2918 : DataStoreWrite  DataStoreName=DSM34
  Block2919 : DataStoreWrite  DataStoreName=DSM29
  wires:
  Block2915/1 -> Block2919/1
  Block2914/1 -> Block2918/1
  Block2913/1 -> Block2917/1

== system lib_all/SubSystem1023/SubSystem1093/SubSystem1106 ==
  note: Text211
  note: Text210
  Block2920 : Inport
  Block2921 : Inport  Port=2
  Block2922 : Inport  Port=3
  Block2923 : Constant  Value=0  OutDataTypeStr=double  SampleTime=-1
  SubSystem1108 : Subsystem
  SubSystem1109 : Subsystem
  SubSystem1117 : Subsystem  TreatAsAtomicUnit=on
  SubSystem1118 : Subsystem
  Block2978 : Outport
  wires:
  Block2921/1 -> [TagName1], SubSystem1109/1(Block2932)
  Block2920/1 -> [TagName4], SubSystem1109/2(Block2933)
  SubSystem1109/1(Block2962) -> [TagName11], SubSystem1118/2(Block2971)
  Block2922/1 -> SubSystem1118/1(Block2970)
  SubSystem1118/1(Block2977) -> [TagName23], Block2978/1
  [TagName4] -> SubSystem1108/1(In1)
  Block2923/1 -> SubSystem1108/2(In2)
  SubSystem1108/1(Out1) -> SubSystem1117/Enable
  [TagName1] -> SubSystem1117/1(Block2963)
  [TagName11] -> SubSystem1117/2(Block2964)
  [TagName23] -> SubSystem1117/3(Block2965)

== system lib_all/SubSystem1023/SubSystem1093/SubSystem1120 ==
  note: Text219
  note: Text218
  note: Text217
  Block2979 : Inport
  Block2980 : Inport  Port=2
  Block2981 : Inport  Port=3
  Block2982 : Constant  OutDataTypeStr=double  SampleTime=-1
  Block2983 : Gain  Gain=1/5  SaturateOnIntegerOverflow=on  OutDataTypeStr=Inherit: Same as input  ParamDataTypeStr=Inherit: Same as input
      settings: AttributesFormatString=Attribute586
  Block2984 : Product  OutDataTypeStr=double  SaturateOnIntegerOverflow=on  RndMeth=Zero  InputSameDT=on
  Block2985 : Sum  Inputs=-+  OutDataTypeStr=double  RndMeth=Zero  SaturateOnIntegerOverflow=on  InputSameDT=on
  Block2986 : Sum  OutDataTypeStr=double  RndMeth=Zero  SaturateOnIntegerOverflow=on  InputSameDT=on
  Block2987 : Sum  OutDataTypeStr=double  RndMeth=Zero  SaturateOnIntegerOverflow=on  InputSameDT=on
  SubSystem1122 : Subsystem
  SubSystem1128 : Subsystem
  Block3078 : Outport
  wires:
  Block2980/1 -> SubSystem1122/1(Block2988)
  SubSystem1122/1(Block3012) -> SubSystem1128/1(Block3014)
  Block2981/1 -> SubSystem1128/2(Block3015)
  SubSystem1128/1(Block3076) -> Block2986/1, Block2985/1
  SubSystem1128/2(Block3077) -> Block2985/2
  Block2985/1 -> Block2984/1  "SigLabel99"
  SubSystem1122/2(Block3013) -> Block2984/2
  Block2986/1 -> Block3078/1
  Block2982/1 -> Block2987/2
  Block2984/1 -> Block2983/1
  Block2983/1 -> Block2986/2
  Block2979/1 -> Block2987/1
  Block2987/1 -> SubSystem1122/2(Block2989), SubSystem1128/3(Block3016)

== system lib_all/SubSystem1023/SubSystem1161/SubSystem1163 ==
  note: ***編集禁止***
  In1 : Inport
  In2 : Inport  Port=2
  RO1 : RelationalOperator  Operator===  InputSameDT=on
  single1 : DataTypeConversion  OutDataTypeStr=single  SaturateOnIntegerOverflow=on  RndMeth=Zero
  single2 : DataTypeConversion  OutDataTypeStr=single  SaturateOnIntegerOverflow=on  RndMeth=Zero
  Out1 : Outport
  wires:
  RO1/1 -> Out1/1
  In2/1 -> single2/1
  In1/1 -> single1/1
  single1/1 -> RO1/1
  single2/1 -> RO1/2

== system lib_all/SubSystem1023/SubSystem1161/SubSystem1164 ==
  note: Text227
  Block3092 : Inport
  Block3093 : Inport  Port=2
  Block3094 : Constant  Value=0  OutDataTypeStr=double  SampleTime=-1
  Block3095 : Constant  Value=720  OutDataTypeStr=double  SampleTime=-1
  Block3096 : Gain  Gain=5  SaturateOnIntegerOverflow=on  OutDataTypeStr=Inherit: Same as input  ParamDataTypeStr=Inherit: Same as input
      settings: AttributesFormatString=Attribute604
  SubSystem1165 : Subsystem
      settings: RTWFcnNameOpts=Use subsystem name
  SubSystem1170 : Subsystem
      settings: RTWSystemCode=Inline
  Block3122 : Outport
  wires:
  Block3096/1 -> SubSystem1170/2(Block3117)
  SubSystem1165/1(Block3115) -> Block3096/1
  Block3093/1 -> SubSystem1165/2(Block3098)
  Block3092/1 -> SubSystem1165/1(Block3097)
  SubSystem1170/1(Block3121) -> Block3122/1
  Block3095/1 -> SubSystem1170/1(Block3116)
  Block3094/1 -> SubSystem1170/3(Block3118)

== system lib_all/SubSystem1023/SubSystem1161/SubSystem1172 ==
  Block3123 : Inport
  Block3124 : Inport  Port=2
  Block3125 : Inport  Port=3
  Block3126 : EnablePort
  Block3127 : DataStoreWrite  DataStoreName=DSM12
  Block3128 : DataStoreWrite  DataStoreName=DSM14
  Block3129 : DataStoreWrite  DataStoreName=DSM13
  wires:
  Block3123/1 -> Block3127/1
  Block3125/1 -> Block3129/1
  Block3124/1 -> Block3128/1

== system lib_all/SubSystem1023/SubSystem1161/SubSystem1173 ==
  note: Text228
  Block3130 : Inport
  Block3131 : Inport  Port=2
  Block3132 : Constant  Value=360  OutDataTypeStr=double  SampleTime=-1
  Block3133 : Constant  Value=360  OutDataTypeStr=double  SampleTime=-1
  Block3134 : Sum  Inputs=+-  OutDataTypeStr=double  RndMeth=Zero  SaturateOnIntegerOverflow=on  InputSameDT=on
  Block3135 : Switch  Threshold=0.5  RndMeth=Zero  SaturateOnIntegerOverflow=on  InputSameDT=on
  Block3136 : Lookup2D  RowIndex=map_lib_05  ColumnIndex=map_lib_04  Table=map_lib_06  SaturateOnIntegerOverflow=on  InputSameDT=on
      settings: AttributesFormatString=<%<OutputValues>>\nAttribute613
  SubSystem1174 : Subsystem
  Block3137 : Outport
  wires:
  Block3131/1 -> SubSystem1174/1(In1), Block3135/3, Block3134/1
  Block3133/1 -> SubSystem1174/2(In2)
  SubSystem1174/1(Out1) -> Block3135/2
  Block3134/1 -> Block3135/1
  Block3132/1 -> Block3134/2
  Block3136/1 -> Block3137/1
  Block3130/1 -> Block3136/2
  Block3135/1 -> Block3136/1  "SigLabel106"

== system lib_all/SubSystem1023/SubSystem1175/SubSystem1177 ==
  note: ***編集禁止***
  In1 : Inport
  In2 : Inport  Port=2
  RO1 : RelationalOperator  Operator===  InputSameDT=on
  single1 : DataTypeConversion  OutDataTypeStr=single  SaturateOnIntegerOverflow=on  RndMeth=Zero
  single2 : DataTypeConversion  OutDataTypeStr=single  SaturateOnIntegerOverflow=on  RndMeth=Zero
  Out1 : Outport
  wires:
  RO1/1 -> Out1/1
  In2/1 -> single2/1
  In1/1 -> single1/1
  single1/1 -> RO1/1
  single2/1 -> RO1/2

== system lib_all/SubSystem1023/SubSystem1175/SubSystem1178 ==
  note: Text231
  Block3151 : Inport
  Block3152 : Inport  Port=2
  Block3153 : Constant  Value=0  OutDataTypeStr=double  SampleTime=-1
  Block3154 : Constant  Value=720  OutDataTypeStr=double  SampleTime=-1
  Block3155 : Gain  Gain=5  SaturateOnIntegerOverflow=on  OutDataTypeStr=Inherit: Same as input  ParamDataTypeStr=Inherit: Same as input
      settings: AttributesFormatString=Attribute618
  SubSystem1179 : Subsystem
      settings: RTWFcnNameOpts=Use subsystem name
  SubSystem1184 : Subsystem
      settings: RTWSystemCode=Inline
  Block3181 : Outport
  wires:
  Block3155/1 -> SubSystem1184/2(Block3176)
  SubSystem1179/1(Block3174) -> Block3155/1
  Block3152/1 -> SubSystem1179/2(Block3157)
  Block3151/1 -> SubSystem1179/1(Block3156)
  SubSystem1184/1(Block3180) -> Block3181/1
  Block3154/1 -> SubSystem1184/1(Block3175)
  Block3153/1 -> SubSystem1184/3(Block3177)

== system lib_all/SubSystem1023/SubSystem1175/SubSystem1186 ==
  Block3182 : Inport
  Block3183 : Inport  Port=2
  Block3184 : Inport  Port=3
  Block3185 : EnablePort
  Block3186 : DataStoreWrite  DataStoreName=DSM12
  Block3187 : DataStoreWrite  DataStoreName=DSM14
  Block3188 : DataStoreWrite  DataStoreName=DSM13
  wires:
  Block3182/1 -> Block3186/1
  Block3184/1 -> Block3188/1
  Block3183/1 -> Block3187/1

== system lib_all/SubSystem1023/SubSystem1175/SubSystem1187 ==
  note: Text232
  Block3189 : Inport
  Block3190 : Inport  Port=2
  Block3191 : Constant  Value=360  OutDataTypeStr=double  SampleTime=-1
  Block3192 : Constant  Value=360  OutDataTypeStr=double  SampleTime=-1
  Block3193 : Sum  Inputs=+-  OutDataTypeStr=double  RndMeth=Zero  SaturateOnIntegerOverflow=on  InputSameDT=on
  Block3194 : Switch  Threshold=0.5  RndMeth=Zero  SaturateOnIntegerOverflow=on  InputSameDT=on
  Block3195 : Lookup2D  RowIndex=map_lib_05  ColumnIndex=map_lib_04  Table=map_lib_06  SaturateOnIntegerOverflow=on  InputSameDT=on
      settings: AttributesFormatString=<%<OutputValues>>\nAttribute627
  SubSystem1188 : Subsystem
  Block3196 : Outport
  wires:
  Block3190/1 -> SubSystem1188/1(In1), Block3194/3, Block3193/1
  Block3192/1 -> SubSystem1188/2(In2)
  SubSystem1188/1(Out1) -> Block3194/2
  Block3193/1 -> Block3194/1
  Block3191/1 -> Block3193/2
  Block3195/1 -> Block3196/1
  Block3189/1 -> Block3195/2
  Block3194/1 -> Block3195/1  "SigLabel107"

== system lib_all/SubSystem1023/SubSystem1189/SubSystem1190 ==
  note: ***編集禁止***
  In1 : Inport
  In2 : Inport  Port=2
  RO1 : RelationalOperator  Operator===  InputSameDT=on
  single1 : DataTypeConversion  OutDataTypeStr=single  SaturateOnIntegerOverflow=on  RndMeth=Zero
  single2 : DataTypeConversion  OutDataTypeStr=single  SaturateOnIntegerOverflow=on  RndMeth=Zero
  Out1 : Outport
  wires:
  RO1/1 -> Out1/1
  In2/1 -> single2/1
  In1/1 -> single1/1
  single1/1 -> RO1/1
  single2/1 -> RO1/2

== system lib_all/SubSystem1023/SubSystem1189/SubSystem1191 ==
  note: ***編集禁止***
  In1 : Inport
  In2 : Inport  Port=2
  RO1 : RelationalOperator  Operator===  InputSameDT=on
  single1 : DataTypeConversion  OutDataTypeStr=single  SaturateOnIntegerOverflow=on  RndMeth=Zero
  single2 : DataTypeConversion  OutDataTypeStr=single  SaturateOnIntegerOverflow=on  RndMeth=Zero
  Out1 : Outport
  wires:
  RO1/1 -> Out1/1
  In2/1 -> single2/1
  In1/1 -> single1/1
  single1/1 -> RO1/1
  single2/1 -> RO1/2

== system lib_all/SubSystem1023/SubSystem1189/SubSystem1192 ==
  note: ***編集禁止***
  In1 : Inport
  In2 : Inport  Port=2
  RO1 : RelationalOperator  Operator===  InputSameDT=on
  single1 : DataTypeConversion  OutDataTypeStr=single  SaturateOnIntegerOverflow=on  RndMeth=Zero
  single2 : DataTypeConversion  OutDataTypeStr=single  SaturateOnIntegerOverflow=on  RndMeth=Zero
  Out1 : Outport
  wires:
  RO1/1 -> Out1/1
  In2/1 -> single2/1
  In1/1 -> single1/1
  single1/1 -> RO1/1
  single2/1 -> RO1/2

== system lib_all/SubSystem1023/SubSystem1189/SubSystem1193 ==
  note: ***編集禁止***
  In1 : Inport
  In2 : Inport  Port=2
  RO1 : RelationalOperator  Operator===  InputSameDT=on
  single1 : DataTypeConversion  OutDataTypeStr=single  SaturateOnIntegerOverflow=on  RndMeth=Zero
  single2 : DataTypeConversion  OutDataTypeStr=single  SaturateOnIntegerOverflow=on  RndMeth=Zero
  Out1 : Outport
  wires:
  RO1/1 -> Out1/1
  In2/1 -> single2/1
  In1/1 -> single1/1
  single1/1 -> RO1/1
  single2/1 -> RO1/2
